simulink model "ofdm_txrx_mimo_2x"  (Simulink 6.6)
settings: Solver=VariableStepDiscrete  StartTime=0.0  StopTime=20e3  RelTol=1e-3
PreLoadFcn:
  | ofdm_rx_mimo_init
InitFcn:
  | ofdm_rx_mimo_init
StartFcn:
  | ofdm_rx_mimo_init
values from neighbouring files (symbols this model uses):
  BER_RAM_init_size = 2048    [ofdm_tx_mimo_init.m]
  CPLength = 16    [ofdm_tx_mimo_init.m]
  CRCPolynomial = hex2dec('04c11db7')    [ofdm_tx_mimo_init.m]
  freqOffset_track_filtCoef_i = hex2dec('2500')/2^32    [ofdm_rx_mimo_init.m]
  freqOffset_track_filtCoef_p = hex2dec('d0000')/2^32    [ofdm_rx_mimo_init.m]
  hi = zeros(1,length(cci))    [ofdm_rx_mimo_init.m]
  hr = zeros(1,length(ccr))    [ofdm_rx_mimo_init.m]
  max_CP_length = 16    [ofdm_tx_mimo_init.m]
  max_num_baseRateSymbols = 31    [ofdm_tx_mimo_init.m]
  max_num_subcarriers = 64    [ofdm_tx_mimo_init.m]
  max_num_trainingSymbols = 15    [ofdm_tx_mimo_init.m]
  max_numBytes = 16384    [ofdm_tx_mimo_init.m]
  max_OFDM_symbols = 2047    [ofdm_tx_mimo_init.m]
  modConstellation_qam16 = .75*[1 3 -1 -3]./3    [ofdm_tx_mimo_init.m]
  modConstellation_qam256 = 0.9375*[3 1 5 7 11 9 13 15 -3 -1 -5 -7 -11 -9 -13 -15]./15    [ofdm_tx_mimo_init.m]
  modConstellation_qam64 = 0.875*[3 1 5 7 -3 -1 -5 -7]./7    [ofdm_tx_mimo_init.m]
  modConstellation_qpsk = [1 -1]./sqrt(2)    [ofdm_tx_mimo_init.m]
  numBaseRateSymbols = 2    [ofdm_tx_mimo_init.m]
  numSubcarriers = 64    [ofdm_tx_mimo_init.m]
  numTrainingSymbols = 2    [ofdm_tx_mimo_init.m]
  pilot1_indicies = 7 + ( (64-7) * 2^16)    (= 3735559)    [ofdm_tx_mimo_init.m]
  pilot2_indicies = 21 + ( (64-21) * 2^16)    (= 2818069)    [ofdm_tx_mimo_init.m]
  pilotValue_neg = hex2dec('8000') + (2^16 * 0)    [ofdm_tx_mimo_init.m]
  pilotValue_pos = hex2dec('7FFF') + (2^16 * 0)    [ofdm_tx_mimo_init.m]
  pkt_crossCorr_thresh = 0.7    [ofdm_rx_mimo_init.m]
  pkt_energy_thresh = 0    [ofdm_rx_mimo_init.m]
  pkt_numPayloadBytes = 1400+42-6    (= 1436)    [ofdm_tx_mimo_init.m]
  pktByteNums = numHeaderBytes +  				byteIndex_numPayloadBytes(1)*2^8 +  				byteIndex_numPayloadBytes(2)*2^16 +  				byteIndex_simpleDynModMasks * 2^24    [ofdm_rx_mimo_init.m]
  PktDet_Delay = 26 + Symbol_Timing_Offset*2^7    (= 1050)    [ofdm_rx_mimo_init.m]
  preamble = 6*[0 shortsyms_10 longsyms_2]    [ofdm_tx_mimo_init.m]
  preamble_I = real(preamble)    [ofdm_tx_mimo_init.m]
  preamble_Q = imag(preamble)    [ofdm_tx_mimo_init.m]
  RAM_init_size = max_numBytes    (= 16384)    [ofdm_tx_mimo_init.m]
  reg_Interrupt_PktBuf_Control = reg_InterruptControl +  	1 * 2^16 +  	0 * 2^24    (= 65656)    [ofdm_rx_mimo_init.m]
  rx_controlBits = 1 * 2  		 + 1 * 4  		 + 0 * 8  		 + 1 * 16 * rx_SISO_Mode  		 + 1 * 32  		 + 1 * 64  		 + 0 * 128  		 + 1 * 256  		 + 0 * 512  		 + 1 * 1024  		 + 0 * 2048  		 + 1 * 4096  		 + 1 * 8192  		 + 0 * 16384  		 + 1 * 65536  		 + 1 * 131072  		 + 0 * 262144  		 + 1 * 524288    [ofdm_rx_mimo_init.m]
  Rx_PhaseNoiseTrack_K = 0.6094    [ofdm_rx_mimo_init.m]
  rx_postEq_scaling = round(rxScaling*2^11) * (1 + 2^16)    [ofdm_rx_mimo_init.m]
  RxDataScrambling_Seq = [40 198 78 63 82 173 102 245 48 111 172 115 147 230 216 93 72 65 62 2 205 242 122 90 128 83 105 97 73 10 5 252]    [ofdm_tx_mimo_init.m]
  simOnly_numSamples = length(preamble)+( (numSubcarriers+CPLength)*(numTrainingSymbols + numBaseRateSymbols + numFullRateSymbols/2) )    [ofdm_tx_mimo_init.m]
  train = [train train]    [ofdm_tx_mimo_init.m]
  tx_controlBits = (antB_preambleShift * 2^4) + (2^0 * tx_SISO_Mode) + 4*0 + 8*1    (= 73)    [ofdm_tx_mimo_init.m]
  TxDataScrambling_Seq = [40 198 78 63 82 173 102 245 48 111 172 115 147 230 216 93 72 65 62 2 205 242 122 90 128 83 105 97 73 10 5 252]    [ofdm_tx_mimo_init.m]
  txReg_symbolCounts = (2^16 * numFullRateSymbols) + (2^8 *numBaseRateSymbols ) + numTrainingSymbols    [ofdm_tx_mimo_init.m]
  TxRx_FFTScaling = bin2dec('010111') + (bin2dec('000101') * 2^6)    [ofdm_tx_mimo_init.m]
undefined symbols (not defined in the file or in its folder): AntA, AntB, BaseRate, CRC_table_gen, gcbh, intfilt, pktTiming_count_payloadStart, pktTiming_symbol_length, xlBlockLoadCallback, xlGetSimulinkPeriod
libraries: xbsIndex_r4 x3973, dspsigops x4, commchan3 x2, commrflib2 x2, dspsrcs4 x1
(part 16 of 38)

== system ofdm_txrx_mimo_2x/OFDM Rx MIMO/Equalizer & Packetizer/Pilot_Extractor/Phase Calc ==
  I : Inport
  Q : Inport  Port=2
  CORDIC ATAN : Subsystem  mask(Xilinx CORDIC ATAN){stages=11, pe_nbits=19, pe_binpt=15, pipeline_x=ones(1,11), prev_stages=11, pipeline=[1  1  1  1  1  1  1  1  1  1  1]}
      mask prompts: stages "Number of Processing Elements (integer value starting from 1)"; pe_nbits "X, Y Data Width"; pe_binpt "X, Y Binary Point Position"; pipeline_x "Latency for each Processing Element [1 0 0 1]"; prev_stages "Previous Number of Stages"; pipeline "Previous Pipeline Values"
      mask help: A parallel implementation, circular vectoring mode CORDIC processor for performing rectangular-to-polar conversion. The number of iteration stages (and resultant output accuracy) and the processor arithmetic precision are controlled by the mask customization.
      mask init: same as ofdm_txrx_mimo_2x/OFDM Rx MIMO/Coarse Freq Correction/Coarse Freq Offset Calc Combo/Arctan2/CORDIC ATAN1
      settings: CopyFcn=set_param(gcb, 'MaskSelfModifiable', 'on', 'LinkStatus', 'none');
      mask Help: eval('xlWeb(xlhtmldoclink(''CORDIC ATAN''))');
      mask Display:
      | patch([0 iWidth iWidth 0],[0 0 iHeight iHeight] , bg);
      | patch(logoX,logoY , fg);
      | text(iCx-6,iCy,'z');
      | text(iCx,iCy+4,str);
      | port_label('input',1,'x');
      | port_label('input',2,'y');
      | port_label('output',1,'mag');
      | port_label('output',2,'atan');
      | plot([0 0 iWidth iWidth 0], [0 iHeight iHeight 0 0]);
  Down Sample : xbsIndex_r4/Down Sample  sample_ratio=2  sample_phase="Last Value of Frame  (most efficient)"  en=off  latency=1  dbl_ovrd=off  block_type=dsamp
      settings: block_version=8.2.02
  Mux : xbsIndex_r4/Mux  inputs=2  en=off  latency=0  precision=Full  arith_type="Signed  (2's comp)"  n_bits=8  bin_pt=2  quantization=Truncate  overflow=Wrap  dbl_ovrd=off  block_type=mux
      settings: xl_area=[16 0 0 32 0 0 0]  block_version=VER_STRING_GOES_HERE
  Up Sample : xbsIndex_r4/Up Sample  sample_ratio=2  copy_samples=on  dbl_ovrd=off  block_type=usamp
      settings: infoedit="Up samples input data.  Inserted values  can be zeros or copies of the most recent input sample.<P><P>Hardware notes: No hardware is needed if inserted values are copies of the input sample; otherwise, a mux and single bit flip-flop are used."  block_version=8.2.02
  Up Sample1 : xbsIndex_r4/Up Sample  sample_ratio=2  copy_samples=on  dbl_ovrd=off  block_type=usamp
      settings: infoedit="Up samples input data.  Inserted values  can be zeros or copies of the most recent input sample.<P><P>Hardware notes: No hardware is needed if inserted values are copies of the input sample; otherwise, a mux and single bit flip-flop are used."  block_version=8.2.02
  Phase : Outport
  wires:
  Up Sample/1 -> CORDIC ATAN/1(x)
  CORDIC ATAN/2(Z) -> Down Sample/1
  Mux/1 -> Phase/1
  [regRx_usePilotArctan] -> Mux/1
  I/1 -> Up Sample/1
  Q/1 -> Mux/2, Up Sample1/1
  Down Sample/1 -> Mux/3
  Up Sample1/1 -> CORDIC ATAN/2(y)

== system ofdm_txrx_mimo_2x/OFDM Rx MIMO/Equalizer & Packetizer/Pilot_Extractor/Pilot_Descrambler ==
  Sym Index : Inport
  rst : Inport  Port=2
  "I " : Inport  Port=3
  "Q " : Inport  Port=4
  FFT Index : Inport  Port=5
  Neg Pilot : Inport  Port=6
  Constant5 : xbsIndex_r4/Constant  arith_type=Unsigned  const=numSubcarriers-1  n_bits=ceil(log2(numSubcarriers))  bin_pt=0  explicit_period=off  period=2  equ=P=C  opselect=C  inp2=PCIN>>17  opr=+  inp1=P  carry=CIN  dbl_ovrd=off  block_type=constant
      settings: block_version=VER_STRING_GOES_HERE
  Gateway Out{1..6} (6 alike) : xbsIndex_r4/Gateway Out  hdl_port=off  timing_constraint=None  locs_specified=off  LOCs={}  block_type=gatewayout
      settings: infoedit="Gateway out block.  Converts Xilinx fixed point inputs into ouputs of type Simulink integer, double, or fixed point.<P><P>Hardware notes:  In hardware these blocks become top level output ports or are discarded, depending on how they are configured."  block_version=VER_STRING_GOES_HERE
  Inverter : xbsIndex_r4/Inverter  en=off  latency=0  dbl_ovrd=off  block_type=inv
      settings: block_version=VER_STRING_GOES_HERE
  LSB : xbsIndex_r4/Slice  nbits=1  boolean_output=on  mode=Lower Bit Location + Width  bit1=0  base1=MSB of Input  bit0=0  base0=LSB of Input  dbl_ovrd=off  block_type=slice
      settings: infoedit="Extracts a given range of bits from each input sample and presents it at the output.  The output type is ordinarily unsigned with binary point at zero, but can be Boolean when the slice is one bit wide.<P><P>Hardware notes: In hardware this block costs nothing."  block_version=VER_STRING_GOES_HERE
  Logical : xbsIndex_r4/Logical  logical_function=XOR  inputs=2  en=off  latency=0  precision=Full  arith_type=Unsigned  n_bits=8  bin_pt=2  align_bp=on  dbl_ovrd=off  block_type=logical
      settings: xl_area=[2 0 0 2 0 0 0]  block_version=VER_STRING_GOES_HERE
  Logical1 : xbsIndex_r4/Logical  logical_function=AND  inputs=3  en=off  latency=0  precision=Full  arith_type=Unsigned  n_bits=8  bin_pt=2  align_bp=on  dbl_ovrd=off  block_type=logical
      settings: xl_area=[2 0 0 2 0 0 0]  block_version=VER_STRING_GOES_HERE
  Mux : xbsIndex_r4/Mux  inputs=2  en=off  latency=0  precision=Full  arith_type="Signed  (2's comp)"  n_bits=8  bin_pt=2  quantization=Truncate  overflow=Wrap  dbl_ovrd=off  block_type=mux
      settings: xl_area=[16 0 0 32 0 0 0]  block_version=VER_STRING_GOES_HERE
  Mux1 : xbsIndex_r4/Mux  inputs=2  en=off  latency=0  precision=Full  arith_type="Signed  (2's comp)"  n_bits=8  bin_pt=2  quantization=Truncate  overflow=Wrap  dbl_ovrd=off  block_type=mux
      settings: xl_area=[16 0 0 32 0 0 0]  block_version=VER_STRING_GOES_HERE
  Negate : xbsIndex_r4/Negate  precision=User Defined  arith_type="Signed  (2's comp)"  n_bits=17  bin_pt=15  quantization="Round  (unbiased: +/- Inf)"  overflow=Saturate  en=off  latency=0  dbl_ovrd=off  block_type=negate
      settings: block_version=VER_STRING_GOES_HERE
  Negate1 : xbsIndex_r4/Negate  precision=User Defined  arith_type="Signed  (2's comp)"  n_bits=17  bin_pt=15  quantization="Round  (unbiased: +/- Inf)"  overflow=Saturate  en=off  latency=0  dbl_ovrd=off  block_type=negate
      settings: block_version=VER_STRING_GOES_HERE
  Pilot Scrambling Sequence Gen : Subsystem
  Relational2 : xbsIndex_r4/Relational  mode=a=b  en=off  latency=0  dbl_ovrd=off  block_type=relational
      settings: xl_area=[6 0 0 11 0 0 0]  block_version=VER_STRING_GOES_HERE
  Relational3 : xbsIndex_r4/Relational  mode=a>=b  en=off  latency=0  dbl_ovrd=off  block_type=relational
      settings: xl_area=[6 0 0 11 0 0 0]  block_version=VER_STRING_GOES_HERE
  Rx Pilot Descrambling : Scope  NumInputPorts=6
      settings: YMin=-1~0.95~0.95~-1~-0.1~-0.075  YMax=1~1.05~1.05~1~0.1~0.1  SaveName=ScopeData41
  S-R Latch : Subsystem
  Shift : xbsIndex_r4/Shift  shift_dir=Left  shift_bits=1  en=off  latency=0  precision=User Defined  arith_type=Unsigned  n_bits=1+ceil(log2(max_num_trainingSymbols))  bin_pt=0  quantization=Truncate  overflow=Flag as error  dbl_ovrd=off  block_type=shift
      settings: block_version=VER_STRING_GOES_HERE
  posedge : Subsystem
  posedge1 : Subsystem
  I : Outport
  Q : Outport  Port=2
  wires:
  Constant5/1 -> Relational2/1
  rst/1 -> Pilot Scrambling Sequence Gen/2(rst), Gateway Out5/1, S-R Latch/2(R)
  "I "/1 -> Mux/2, Gateway Out6/1, Negate/1
  "Q "/1 -> Negate1/1, Mux1/2
  Pilot Scrambling Sequence Gen/1(Q) -> Gateway Out3/1, Logical/1
  Neg Pilot/1 -> Logical/2
  FFT Index/1 -> Relational2/2
  Mux/1 -> I/1, Gateway Out1/1
  Mux1/1 -> Q/1
  Sym Index/1 -> Relational3/1, LSB/1
  Shift/1 -> Relational3/2
  Logical1/1 -> Pilot Scrambling Sequence Gen/1(en), Gateway Out2/1
  Relational2/1 -> posedge1/1(D)
  Relational3/1 -> Gateway Out4/1, posedge/1(D)
  Gateway Out5/1 -> Rx Pilot Descrambling/1
  Gateway Out4/1 -> Rx Pilot Descrambling/2
  Gateway Out3/1 -> Rx Pilot Descrambling/3
  Gateway Out2/1 -> Rx Pilot Descrambling/4
  [regRx_numTrainingSymbols] -> Shift/1
  Logical/1 -> Mux1/1, Mux/1
  Gateway Out6/1 -> Rx Pilot Descrambling/5
  Gateway Out1/1 -> Rx Pilot Descrambling/6
  posedge/1(Q) -> S-R Latch/1(S)
  S-R Latch/1(D) -> Logical1/3
  Negate/1 -> Mux/3
  Negate1/1 -> Mux1/3
  LSB/1 -> Inverter/1
  Inverter/1 -> Logical1/1
  posedge1/1(Q) -> Logical1/2

== system ofdm_txrx_mimo_2x/OFDM Rx MIMO/Equalizer & Packetizer/Pilot_Extractor/S-R Latch ==
  S : Inport
  R : Inport  Port=2
  Constant2 : xbsIndex_r4/Constant  arith_type=Boolean  const=1  n_bits=1  bin_pt=0  explicit_period=off  period=1  equ=P=C  opselect=C  inp2=PCIN>>17  opr=+  inp1=P  carry=CIN  dbl_ovrd=off  block_type=constant
      settings: block_version=VER_STRING_GOES_HERE
  Register : xbsIndex_r4/Register  init=0  rst=on  en=on  dbl_ovrd=off  block_type=register
      settings: xl_area=[0, 0, 0, 0, 0, 0, 0]  block_version=VER_STRING_GOES_HERE
  D : Outport
  wires:
  Constant2/1 -> Register/1
  R/1 -> Register/2
  S/1 -> Register/3
  Register/1 -> D/1

== system ofdm_txrx_mimo_2x/OFDM Rx MIMO/Equalizer & Packetizer/Pilot_Extractor/posedge ==
  D : Inport  Orientation=down
  Inverter : xbsIndex_r4/Inverter  en=off  latency=1  dbl_ovrd=off  block_type=inv
      settings: block_version=VER_STRING_GOES_HERE
  Logical1 : xbsIndex_r4/Logical  logical_function=AND  inputs=2  en=off  latency=0  precision=Full  arith_type=Unsigned  n_bits=8  bin_pt=2  align_bp=on  dbl_ovrd=off  block_type=logical
      settings: xl_area=[1 0 0 1 0 0 0]  block_version=VER_STRING_GOES_HERE
  Q : Outport
  wires:
  Inverter/1 -> Logical1/2
  D/1 -> Logical1/1, Inverter/1
  Logical1/1 -> Q/1

== system ofdm_txrx_mimo_2x/OFDM Rx MIMO/FFT & Chan Est/Addr Gen1/Copy_Downsample ==
  D : Inport
  Delay : xbsIndex_r4/Delay  en=off  latency=1  dbl_ovrd=off  reg_retiming=off  block_type=delay
      settings: infoedit=Hardware notes: A delay line is a chain, each link of which is an SRL16 followed by a flip-flop. If register retiming is enabled, the delay line is a chain of flip-flops.  block_version=VER_STRING_GOES_HERE
  Down Sample : xbsIndex_r4/Down Sample  sample_ratio=2  sample_phase="Last Value of Frame  (most efficient)"  en=off  latency=1  dbl_ovrd=off  block_type=dsamp
      settings: block_version=VER_STRING_GOES_HERE
  Logical : xbsIndex_r4/Logical  logical_function=OR  inputs=2  en=off  latency=0  precision=Full  arith_type=Unsigned  n_bits=16  bin_pt=0  align_bp=on  dbl_ovrd=off  block_type=logical
      settings: block_version=VER_STRING_GOES_HERE
  Q : Outport
  wires:
  Delay/1 -> Logical/1
  D/1 -> Delay/1, Logical/2
  Logical/1 -> Down Sample/1
  Down Sample/1 -> Q/1

== system ofdm_txrx_mimo_2x/OFDM Rx MIMO/FFT & Chan Est/Addr Gen1/Oscillator ==
  en : Inport
  rst : Inport  Port=2
  Assert : xbsIndex_r4/Assert  Orientation=left  assert_type=on  type_source=Explicitly  arith_type=Boolean  n_bits=16  bin_pt=14  assert_rate=on  rate_source=Explicitly  period=2  output_port=on  block_type=assert
      settings: infoedit=Asserts a user-defined sample rate and/or type on a signal.<P><P>Hardware notes: In hardware this block costs nothing.  block_version=VER_STRING_GOES_HERE
  Inverter1 : xbsIndex_r4/Inverter  Orientation=left  en=off  latency=0  dbl_ovrd=off  block_type=inv
      settings: block_version=VER_STRING_GOES_HERE
  Register : xbsIndex_r4/Register  init=0  rst=on  en=on  dbl_ovrd=off  block_type=register
      settings: block_version=VER_STRING_GOES_HERE
  Q : Outport
  wires:
  Register/1 -> Q/1, Assert/1
  en/1 -> Register/3
  Inverter1/1 -> Register/1
  rst/1 -> Register/2
  Assert/1 -> Inverter1/1

== system ofdm_txrx_mimo_2x/OFDM Rx MIMO/FFT & Chan Est/Addr Gen1/Output Sym Counter ==
  FFT Done : Inport
  Not Training : Inport  Port=2
  Payload : Inport  Port=3
  Read Addr : Inport  Port=4
  Constant1 : xbsIndex_r4/Constant  arith_type=Unsigned  const=numSubcarriers-1  n_bits=ceil(log2(numSubcarriers))  bin_pt=0  explicit_period=off  period=1  equ=P=C  opselect=C  inp2=PCIN>>17  opr=+  inp1=P  carry=CIN  dbl_ovrd=off  block_type=constant
      settings: block_version=VER_STRING_GOES_HERE
  Counter1 : xbsIndex_r4/Counter  cnt_type=Free Running  cnt_to=127  operation=Up  start_count=0  cnt_by_val=1  arith_type=Unsigned  n_bits=ceil(log2(max_OFDM_symbols))  bin_pt=0  load_pin=off  rst=on  en=on  explicit_period=off  period=2  dbl_ovrd=off  use_behavioral_HDL=off  use_rpm=on  implementation=Fabric  block_type=counter
      settings: xl_area=[6 12 0 12 0 0 0]  block_version=VER_STRING_GOES_HERE
  FFT Output Buffers Addrs 3 : Scope  NumInputPorts=6
      settings: TimeRange=4000  YMin=5~0~0~-1~0~0  YMax=13~1~1~1~1~65  SaveName=ScopeData75
  Inverter3 : xbsIndex_r4/Inverter  en=off  latency=0  dbl_ovrd=off  block_type=inv
      settings: block_version=VER_STRING_GOES_HERE
  Logical1 : xbsIndex_r4/Logical  logical_function=AND  inputs=2  en=off  latency=0  precision=Full  arith_type=Unsigned  n_bits=8  bin_pt=2  align_bp=on  dbl_ovrd=off  block_type=logical
      settings: xl_area=[0, 0, 0, 0, 0, 0, 0]  block_version=VER_STRING_GOES_HERE
  Logical2 : xbsIndex_r4/Logical  logical_function=OR  inputs=2  en=off  latency=0  precision=Full  arith_type=Unsigned  n_bits=8  bin_pt=2  align_bp=on  dbl_ovrd=off  block_type=logical
      settings: xl_area=[0, 0, 0, 0, 0, 0, 0]  block_version=VER_STRING_GOES_HERE
  Logical3 : xbsIndex_r4/Logical  logical_function=AND  inputs=2  en=off  latency=0  precision=Full  arith_type=Unsigned  n_bits=8  bin_pt=2  align_bp=on  dbl_ovrd=off  block_type=logical
      settings: xl_area=[0, 0, 0, 0, 0, 0, 0]  block_version=VER_STRING_GOES_HERE
  Logical4 : xbsIndex_r4/Logical  logical_function=AND  inputs=2  en=off  latency=0  precision=Full  arith_type=Unsigned  n_bits=8  bin_pt=2  align_bp=on  dbl_ovrd=off  block_type=logical
      settings: xl_area=[0, 0, 0, 0, 0, 0, 0]  block_version=VER_STRING_GOES_HERE
  Posedge : Subsystem
  Posedge1 : Subsystem
  Posedge2 : Subsystem
  Posedge3 : Subsystem
  Relational : xbsIndex_r4/Relational  mode=a=b  en=off  latency=0  dbl_ovrd=off  block_type=relational
      settings: block_version=VER_STRING_GOES_HERE
  S-R Latch2 : Subsystem
  done{1,9..10,16..18} (6 alike) : xbsIndex_r4/Gateway Out  hdl_port=off  timing_constraint=None  locs_specified=off  LOCs={}  block_type=gatewayout
      settings: infoedit="Gateway out block.  Converts Xilinx fixed point inputs into ouputs of type Simulink integer, double, or fixed point.<P><P>Hardware notes:  In hardware these blocks become top level output ports or are discarded, depending on how they are configured."  xl_area=[0 0 0 0 1 0 0]  block_version=VER_STRING_GOES_HERE
  Sym Index : Outport
  wires:
  Logical4/1 -> Logical3/2
  S-R Latch2/1(D) -> Logical4/2
  Posedge/1(Q) -> S-R Latch2/1(S), Logical4/1, done16/1
  FFT Done/1 -> Posedge/1(D)
  Counter1/1 -> Sym Index/1, done9/1
  Not Training/1 -> Inverter3/1, Logical1/2
  Inverter3/1 -> Logical3/1
  Payload/1 -> Posedge1/1(D)
  Posedge1/1(Q) -> S-R Latch2/2(R), Counter1/1
  Read Addr/1 -> Relational/2, done1/1
  Constant1/1 -> Relational/1
  Relational/1 -> Logical1/1
  Logical1/1 -> Posedge2/1(D)
  Logical3/1 -> Posedge3/1(D)
  Logical2/1 -> Counter1/2, done10/1
  done16/1 -> FFT Output Buffers Addrs 3/5
  done9/1 -> FFT Output Buffers Addrs 3/1
  done10/1 -> FFT Output Buffers Addrs 3/2
  done18/1 -> FFT Output Buffers Addrs 3/3
  done17/1 -> FFT Output Buffers Addrs 3/4
  done1/1 -> FFT Output Buffers Addrs 3/6
  Posedge2/1(Q) -> Logical2/1, done18/1
  Posedge3/1(Q) -> Logical2/2, done17/1

== system ofdm_txrx_mimo_2x/OFDM Rx MIMO/FFT & Chan Est/Addr Gen1/S-R Latch1 ==
  S : Inport
  R : Inport  Port=2
  Constant2 : xbsIndex_r4/Constant  arith_type=Boolean  const=1  n_bits=1  bin_pt=0  explicit_period=off  period=1  equ=P=C  opselect=C  inp2=PCIN>>17  opr=+  inp1=P  carry=CIN  dbl_ovrd=off  block_type=constant
      settings: block_version=VER_STRING_GOES_HERE
  Register : xbsIndex_r4/Register  init=0  rst=on  en=on  dbl_ovrd=off  block_type=register
      settings: xl_area=[0, 0, 0, 0, 0, 0, 0]  block_version=VER_STRING_GOES_HERE
  D : Outport
  wires:
  Register/1 -> D/1
  S/1 -> Register/3
  R/1 -> Register/2
  Constant2/1 -> Register/1

== system ofdm_txrx_mimo_2x/OFDM Rx MIMO/FFT & Chan Est/Addr_Generator/Initial Read Addr Timing Calc ==
  note: The FFT runs 4x as fast as the input samples. As the input
      | samples are written to the buffers, the FFT waits to start
      | until it can have access to the newest sample it needs
      | 1 cycle after it's written.
  AddSub1 : xbsIndex_r4/AddSub  mode=Addition  use_carryin=off  use_carryout=off  en=off  latency=0  precision=User Defined  arith_type=Unsigned  n_bits=ceil(log2(max_num_subcarriers))+1  bin_pt=0  quantization=Truncate  overflow=Wrap  dbl_ovrd=off  use_behavioral_HDL=on  pipelined=off  use_rpm=on  hw_selection=Fabric  block_type=addsub
      settings: xl_area=[4 0 0 6 0 0 0]  block_version=VER_STRING_GOES_HERE
  Constant1 : xbsIndex_r4/Constant  arith_type=Unsigned  const=0.75*numSubcarriers  n_bits=log2(numSubcarriers)  bin_pt=0  explicit_period=off  period=1  equ=P=C  opselect=C  inp2=PCIN>>17  opr=+  inp1=P  carry=CIN  dbl_ovrd=off  block_type=constant
      settings: block_version=VER_STRING_GOES_HERE
  Display : Display
  Display1 : Display
  Display3 : Display
  Up Sample4 : xbsIndex_r4/Up Sample  sample_ratio=2  copy_samples=on  dbl_ovrd=off  block_type=usamp
      settings: infoedit="Up samples input data.  Inserted values  can be zeros or copies of the most recent input sample.<P><P>Hardware notes: No hardware is needed if inserted values are copies of the input sample; otherwise, a mux and single bit flip-flop are used."  block_version=VER_STRING_GOES_HERE
  done2 : xbsIndex_r4/Gateway Out  hdl_port=off  timing_constraint=None  locs_specified=off  LOCs={}  block_type=gatewayout
      settings: infoedit="Gateway out block.  Converts Xilinx fixed point inputs into ouputs of type Simulink integer, double, or fixed point.<P><P>Hardware notes:  In hardware these blocks become top level output ports or are discarded, depending on how they are configured."  xl_area=[0 0 0 0 1 0 0]  block_version=VER_STRING_GOES_HERE
  done7 : xbsIndex_r4/Gateway Out  hdl_port=off  timing_constraint=None  locs_specified=off  LOCs={}  block_type=gatewayout
      settings: infoedit="Gateway out block.  Converts Xilinx fixed point inputs into ouputs of type Simulink integer, double, or fixed point.<P><P>Hardware notes:  In hardware these blocks become top level output ports or are discarded, depending on how they are configured."  xl_area=[0 0 0 0 1 0 0]  block_version=VER_STRING_GOES_HERE
  done9 : xbsIndex_r4/Gateway Out  hdl_port=off  timing_constraint=None  locs_specified=off  LOCs={}  block_type=gatewayout
      settings: infoedit="Gateway out block.  Converts Xilinx fixed point inputs into ouputs of type Simulink integer, double, or fixed point.<P><P>Hardware notes:  In hardware these blocks become top level output ports or are discarded, depending on how they are configured."  xl_area=[0 0 0 0 1 0 0]  block_version=VER_STRING_GOES_HERE
  D : Outport
  wires:
  AddSub1/1 -> done2/1, D/1
  done9/1 -> Display3/1
  done7/1 -> Display1/1
  done2/1 -> Display/1
  [regRx_symbolTimingOffset] -> Up Sample4/1
  Up Sample4/1 -> done9/1, AddSub1/2
  Constant1/1 -> done7/1, AddSub1/1

== system ofdm_txrx_mimo_2x/OFDM Rx MIMO/FFT & Chan Est/Addr_Generator/Posedge ==
  D : Inport
  Delay : xbsIndex_r4/Delay  en=off  latency=1  dbl_ovrd=off  reg_retiming=off  block_type=delay
      settings: infoedit=Hardware notes: A delay line is a chain, each link of which is an SRL16 followed by a flip-flop. If register retiming is enabled, the delay line is a chain of flip-flops.  xl_area=[1 1 0 0 0 0 0]  block_version=VER_STRING_GOES_HERE
  Inverter : xbsIndex_r4/Inverter  en=off  latency=0  dbl_ovrd=off  block_type=inv
      settings: xl_area=[0 0 0 0 0 0 0]  block_version=VER_STRING_GOES_HERE
  Logical : xbsIndex_r4/Logical  logical_function=AND  inputs=2  en=off  latency=0  precision=Full  arith_type=Unsigned  n_bits=8  bin_pt=2  align_bp=on  dbl_ovrd=off  block_type=logical
      settings: xl_area=[1 0 0 1 0 0 0]  block_version=VER_STRING_GOES_HERE
  Q : Outport
  wires:
  Logical/1 -> Q/1
  D/1 -> Delay/1, Logical/1
  Inverter/1 -> Logical/2
  Delay/1 -> Inverter/1

== system ofdm_txrx_mimo_2x/OFDM Rx MIMO/FFT & Chan Est/Addr_Generator/negedge1 ==
  D : Inport
  Delay : xbsIndex_r4/Delay  en=off  latency=1  dbl_ovrd=off  reg_retiming=off  block_type=delay
      settings: infoedit=Hardware notes: A delay line is a chain, each link of which is an SRL16 followed by a flip-flop. If register retiming is enabled, the delay line is a chain of flip-flops.  xl_area=[1 1 0 0 0 0 0]  block_version=VER_STRING_GOES_HERE
  Inverter : xbsIndex_r4/Inverter  en=off  latency=0  dbl_ovrd=off  block_type=inv
      settings: xl_area=[0 0 0 0 0 0 0]  block_version=VER_STRING_GOES_HERE
  Logical : xbsIndex_r4/Logical  logical_function=AND  inputs=2  en=off  latency=0  precision=Full  arith_type=Unsigned  n_bits=8  bin_pt=2  align_bp=on  dbl_ovrd=off  block_type=logical
      settings: xl_area=[1 0 0 1 0 0 0]  block_version=VER_STRING_GOES_HERE
  Q : Outport
  wires:
  Inverter/1 -> Logical/1
  Delay/1 -> Logical/2
  D/1 -> Inverter/1, Delay/1
  Logical/1 -> Q/1

== system ofdm_txrx_mimo_2x/OFDM Rx MIMO/FFT & Chan Est/Channel Estimation/Bus Creator1 ==
  AA-I : Inport
  AA-Q : Inport  Port=2
  AB-I : Inport  Port=3
  AB-Q : Inport  Port=4
  BA-I : Inport  Port=5
  BA-Q : Inport  Port=6
  BB-I : Inport  Port=7
  BB-Q : Inport  Port=8
  Bus Creator1 : BusCreator
  Bus Creator2 : BusCreator
  I : Outport
  Q : Outport  Port=2
  wires:
  AA-I/1 -> Bus Creator1/1
  AB-I/1 -> Bus Creator1/2
  Bus Creator1/1 -> I/1
  BA-I/1 -> Bus Creator1/3
  BB-I/1 -> Bus Creator1/4
  AA-Q/1 -> Bus Creator2/1
  AB-Q/1 -> Bus Creator2/2
  Bus Creator2/1 -> Q/1
  BA-Q/1 -> Bus Creator2/3
  BB-Q/1 -> Bus Creator2/4

== system ofdm_txrx_mimo_2x/OFDM Rx MIMO/FFT & Chan Est/Channel Estimation/Downsamplng ==
  AA-I : Inport
  AA-Q : Inport  Port=2
  AB-I : Inport  Port=3
  AB-Q : Inport  Port=4
  BA-I : Inport  Port=5
  BA-Q : Inport  Port=6
  BB-I : Inport  Port=7
  BB-Q : Inport  Port=8
  Down Sample{,1..7} (8 alike) : xbsIndex_r4/Down Sample  sample_ratio=2  sample_phase="Last Value of Frame  (most efficient)"  en=off  latency=1  dbl_ovrd=off  block_type=dsamp
      settings: block_version=10.1.2
  " AA-I" : Outport
  " AA-Q" : Outport  Port=2
  " AB-I" : Outport  Port=3
  " AB-Q" : Outport  Port=4
  " BA-I" : Outport  Port=5
  " BA-Q" : Outport  Port=6
  " BB-I" : Outport  Port=7
  " BB-Q" : Outport  Port=8
  wires:
  AA-I/1 -> Down Sample/1
  AB-I/1 -> Down Sample1/1
  AA-Q/1 -> Down Sample4/1
  BA-I/1 -> Down Sample2/1
  AB-Q/1 -> Down Sample5/1
  BB-I/1 -> Down Sample3/1
  BA-Q/1 -> Down Sample6/1
  BB-Q/1 -> Down Sample7/1
  Down Sample/1 -> " AA-I"/1
  Down Sample1/1 -> " AB-I"/1
  Down Sample2/1 -> " BA-I"/1
  Down Sample3/1 -> " BB-I"/1
  Down Sample4/1 -> " AA-Q"/1
  Down Sample5/1 -> " AB-Q"/1
  Down Sample6/1 -> " BA-Q"/1
  Down Sample7/1 -> " BB-Q"/1

== system ofdm_txrx_mimo_2x/OFDM Rx MIMO/FFT & Chan Est/Channel Estimation/H_A-A Estimate Buffer ==
  I : Inport
  Q : Inport  Port=2
  Est_WE : Inport  Port=3
  Est_Reset : Inport  Port=4
  Xk_Index : Inport  Port=5
  I Estimate Buffer : Subsystem
  Q Estimate Buffer : Subsystem
  EstI : Outport
  EstQ : Outport  Port=2
  wires:
  Q/1 -> Q Estimate Buffer/1(Est_Data)
  Q Estimate Buffer/1(h[n]) -> EstQ/1
  Est_Reset/1 -> Q Estimate Buffer/4(Rst), I Estimate Buffer/4(Rst)
  Est_WE/1 -> Q Estimate Buffer/3(Est_WE), I Estimate Buffer/3(Est_WE)
  I Estimate Buffer/1(h[n]) -> EstI/1
  Xk_Index/1 -> Q Estimate Buffer/2(Est_Addr), I Estimate Buffer/2(Est_Addr)
  I/1 -> I Estimate Buffer/1(Est_Data)

== system ofdm_txrx_mimo_2x/OFDM Rx MIMO/FFT & Chan Est/Channel Estimation/H_A-B Estimate Buffer ==
  I : Inport
  Q : Inport  Port=2
  Est_WE : Inport  Port=3
  Est_Reset : Inport  Port=4
  Xk_Index : Inport  Port=5
  I Estimate Buffer : Subsystem
  Q Estimate Buffer : Subsystem
  EstI : Outport
  EstQ : Outport  Port=2
  wires:
  I/1 -> I Estimate Buffer/1(Est_Data)
  Xk_Index/1 -> I Estimate Buffer/2(Est_Addr), Q Estimate Buffer/2(Est_Addr)
  I Estimate Buffer/1(h[n]) -> EstI/1
  Est_WE/1 -> I Estimate Buffer/3(Est_WE), Q Estimate Buffer/3(Est_WE)
  Est_Reset/1 -> I Estimate Buffer/4(Rst), Q Estimate Buffer/4(Rst)
  Q Estimate Buffer/1(h[n]) -> EstQ/1
  Q/1 -> Q Estimate Buffer/1(Est_Data)

== system ofdm_txrx_mimo_2x/OFDM Rx MIMO/FFT & Chan Est/Channel Estimation/H_B-A Estimate Buffer ==
  I : Inport
  Q : Inport  Port=2
  Est_WE : Inport  Port=3
  Est_Reset : Inport  Port=4
  Xk_Index : Inport  Port=5
  I Estimate Buffer : Subsystem
  Q Estimate Buffer : Subsystem
  EstI : Outport
  EstQ : Outport  Port=2
  wires:
  I/1 -> I Estimate Buffer/1(Est_Data)
  Xk_Index/1 -> I Estimate Buffer/2(Est_Addr), Q Estimate Buffer/2(Est_Addr)
  I Estimate Buffer/1(h[n]) -> EstI/1
  Est_WE/1 -> I Estimate Buffer/3(Est_WE), Q Estimate Buffer/3(Est_WE)
  Est_Reset/1 -> I Estimate Buffer/4(Rst), Q Estimate Buffer/4(Rst)
  Q Estimate Buffer/1(h[n]) -> EstQ/1
  Q/1 -> Q Estimate Buffer/1(Est_Data)

== system ofdm_txrx_mimo_2x/OFDM Rx MIMO/FFT & Chan Est/Channel Estimation/H_B-B Estimate Buffer ==
  I : Inport
  Q : Inport  Port=2
  Est_WE : Inport  Port=3
  Est_Reset : Inport  Port=4
  Xk_Index : Inport  Port=5
  I Estimate Buffer : Subsystem
  Q Estimate Buffer : Subsystem
  EstI : Outport
  EstQ : Outport  Port=2
  wires:
  Q/1 -> Q Estimate Buffer/1(Est_Data)
  Q Estimate Buffer/1(h[n]) -> EstQ/1
  Est_Reset/1 -> Q Estimate Buffer/4(Rst), I Estimate Buffer/4(Rst)
  Est_WE/1 -> Q Estimate Buffer/3(Est_WE), I Estimate Buffer/3(Est_WE)
  I Estimate Buffer/1(h[n]) -> EstI/1
  Xk_Index/1 -> Q Estimate Buffer/2(Est_Addr), I Estimate Buffer/2(Est_Addr)
  I/1 -> I Estimate Buffer/1(Est_Data)

== system ofdm_txrx_mimo_2x/OFDM Rx MIMO/FFT & Chan Est/Channel Estimation/Mult by training ==
  AR : Inport
  AI : Inport  Port=2
  BR : Inport  Port=3
  Constant1 : xbsIndex_r4/Constant  arith_type=Signed (2's comp)  const=0  n_bits=2  bin_pt=1  explicit_period=off  period=1  equ=P=C  opselect=C  inp2=PCIN>>17  opr=+  inp1=P  carry=CIN  dbl_ovrd=off  block_type=constant
      settings: block_version=VER_STRING_GOES_HERE
  Constant2 : xbsIndex_r4/Constant  arith_type=Signed (2's comp)  const=0  n_bits=2  bin_pt=1  explicit_period=off  period=1  equ=P=C  opselect=C  inp2=PCIN>>17  opr=+  inp1=P  carry=CIN  dbl_ovrd=off  block_type=constant
      settings: block_version=VER_STRING_GOES_HERE
  Mult by Training : Scope  NumInputPorts=5
      settings: TimeRange=5000  YMin=-1~-1~-1~0~0  YMax=1~1~1~1~1  SaveName=ScopeData22
  Mux3 : xbsIndex_r4/Mux  inputs=3  en=off  latency=1  precision=Full  arith_type="Signed  (2's comp)"  n_bits=8  bin_pt=2  quantization=Truncate  overflow=Wrap  dbl_ovrd=off  block_type=mux
      settings: xl_area=[0, 0, 0, 0, 0, 0, 0]  block_version=VER_STRING_GOES_HERE
  Mux4 : xbsIndex_r4/Mux  inputs=3  en=off  latency=1  precision=Full  arith_type="Signed  (2's comp)"  n_bits=8  bin_pt=2  quantization=Truncate  overflow=Wrap  dbl_ovrd=off  block_type=mux
      settings: xl_area=[0, 0, 0, 0, 0, 0, 0]  block_version=VER_STRING_GOES_HERE
  Negate : xbsIndex_r4/Negate  precision=User Defined  arith_type="Signed  (2's comp)"  n_bits=16  bin_pt=15  quantization=Truncate  overflow=Flag as error  en=off  latency=0  dbl_ovrd=off  block_type=negate
      settings: xl_area=[0, 0, 0, 0, 0, 0, 0]  block_version=VER_STRING_GOES_HERE
  Negate1 : xbsIndex_r4/Negate  precision=User Defined  arith_type="Signed  (2's comp)"  n_bits=16  bin_pt=15  quantization=Truncate  overflow=Flag as error  en=off  latency=0  dbl_ovrd=off  block_type=negate
      settings: xl_area=[0, 0, 0, 0, 0, 0, 0]  block_version=VER_STRING_GOES_HERE
  Reinterpret : xbsIndex_r4/Reinterpret  force_arith_type=on  arith_type=Unsigned  force_bin_pt=on  bin_pt=0  block_type=cast
      settings: infoedit="Changes type of samples without altering their binary representation.<P><P>Hardware notes: In hardware this block costs nothing.<P><P>Example:  Suppose the input is 6 bits wide, signed, with 2 fractional bits, and the output is forced to unsigned with 0 fractional bits.  Then an input of -2.0 (1110.00 in binary 2's complement) becomes an output of 56 (111000 in binary)."  block_version=VER_STRING_GOES_HERE
  done{1..2,7,12..13} (5 alike) : xbsIndex_r4/Gateway Out  hdl_port=off  timing_constraint=None  locs_specified=off  LOCs={}  block_type=gatewayout
      settings: infoedit="Gateway out block.  Converts Xilinx fixed point inputs into ouputs of type Simulink integer, double, or fixed point.<P><P>Hardware notes:  In hardware these blocks become top level output ports or are discarded, depending on how they are configured."  xl_area=[0 0 0 0 1 0 0]  block_version=VER_STRING_GOES_HERE
  PR : Outport
  PI : Outport  Port=2
  wires:
  done1/1 -> Mult by Training/5
  done7/1 -> Mult by Training/4
  done2/1 -> Mult by Training/1
  done13/1 -> Mult by Training/3
  done12/1 -> Mult by Training/2
  Reinterpret/1 -> Mux4/1, Mux3/1
  Mux3/1 -> done7/1, PR/1
  Negate/1 -> Mux3/4
  Constant2/1 -> Mux3/2
  Mux4/1 -> done1/1, PI/1
  Negate1/1 -> Mux4/4
  Constant1/1 -> Mux4/2
  AI/1 -> done13/1, Mux4/3, Negate1/1
  BR/1 -> done2/1, Reinterpret/1
  AR/1 -> done12/1, Mux3/3, Negate/1

== system ofdm_txrx_mimo_2x/OFDM Rx MIMO/FFT & Chan Est/Channel Estimation/WE Gen ==
  note: SISOMode=1 writes estimates for antenna A for every
      | training symbol. SISOMode=0 (i.e. MIMO mode) alternates
      | between antenna A and B training symbols.
  Vin : Inport
  Sym Index : Inport  Port=2
  Train : Inport  Port=3
  1LSB : xbsIndex_r4/Slice  nbits=1  boolean_output=on  mode=Lower Bit Location + Width  bit1=0  base1=MSB of Input  bit0=0  base0=LSB of Input  dbl_ovrd=off  block_type=slice
      settings: infoedit="Extracts a given range of bits from each input sample and presents it at the output.  The output type is ordinarily unsigned with binary point at zero, but can be Boolean when the slice is one bit wide.<P><P>Hardware notes: In hardware this block costs nothing."  block_version=VER_STRING_GOES_HERE
  1LSB+1 : xbsIndex_r4/Slice  nbits=1  boolean_output=on  mode=Lower Bit Location + Width  bit1=0  base1=MSB of Input  bit0=1  base0=LSB of Input  dbl_ovrd=off  block_type=slice
      settings: infoedit="Extracts a given range of bits from each input sample and presents it at the output.  The output type is ordinarily unsigned with binary point at zero, but can be Boolean when the slice is one bit wide.<P><P>Hardware notes: In hardware this block costs nothing."  block_version=VER_STRING_GOES_HERE
  Delay{1..4} (4 alike) : xbsIndex_r4/Delay  en=off  latency=1  dbl_ovrd=off  reg_retiming=on  block_type=delay
      settings: infoedit=Hardware notes: A delay line is a chain, each link of which is an SRL16 followed by a flip-flop. If register retiming is enabled, the delay line is a chain of flip-flops.  xl_area=[0, 0, 0, 0, 0, 0, 0]  block_version=VER_STRING_GOES_HERE
  Expression : xbsIndex_r4/Expression  expression=a & b & ~c & ~d  align_bp=on  en=off  latency=0  precision=Full  arith_type=Unsigned  n_bits=16  bin_pt=0  dbl_ovrd=off  block_type=expr
      settings: block_version=VER_STRING_GOES_HERE
  Expression10 : xbsIndex_r4/Expression  expression=a & b & c & d  align_bp=on  en=off  latency=0  precision=Full  arith_type=Unsigned  n_bits=16  bin_pt=0  dbl_ovrd=off  block_type=expr
      settings: block_version=VER_STRING_GOES_HERE
  Expression8 : xbsIndex_r4/Expression  expression=a & b & ~c & d  align_bp=on  en=off  latency=0  precision=Full  arith_type=Unsigned  n_bits=16  bin_pt=0  dbl_ovrd=off  block_type=expr
      settings: block_version=VER_STRING_GOES_HERE
  Expression9 : xbsIndex_r4/Expression  expression=a & b & c & ~d  align_bp=on  en=off  latency=0  precision=Full  arith_type=Unsigned  n_bits=16  bin_pt=0  dbl_ovrd=off  block_type=expr
      settings: block_version=VER_STRING_GOES_HERE
  Inverter : xbsIndex_r4/Inverter  en=off  latency=0  dbl_ovrd=off  block_type=inv
      settings: block_version=VER_STRING_GOES_HERE
  Logical2 : xbsIndex_r4/Logical  logical_function=AND  inputs=2  en=off  latency=0  precision=Full  arith_type=Unsigned  n_bits=8  bin_pt=2  align_bp=on  dbl_ovrd=off  block_type=logical
      settings: xl_area=[1 0 0 1 0 0 0]  block_version=VER_STRING_GOES_HERE
  Seq0 : Outport
  Seq1 : Outport  Port=2
  Seq2 : Outport  Port=3
  Seq3 : Outport  Port=4
  wires:
  Inverter/1 -> Logical2/1
  1LSB+1/1 -> Logical2/2
  [regRx_SISOmode] -> Inverter/1
  Delay4/1 -> Seq3/1
  Delay2/1 -> Seq2/1
  Delay1/1 -> Seq1/1
  Delay3/1 -> Seq0/1
  1LSB/1 -> Expression10/4, Expression9/4, Expression8/4, Expression/4
  Vin/1 -> Expression10/1, Expression9/1, Expression8/1, Expression/1
  Expression10/1 -> Delay4/1
  Expression9/1 -> Delay2/1
  Expression8/1 -> Delay1/1
  Expression/1 -> Delay3/1
  Logical2/1 -> Expression/3, Expression8/3, Expression9/3, Expression10/3
  Sym Index/1 -> 1LSB/1, 1LSB+1/1
  Train/1 -> Expression/2, Expression8/2, Expression9/2, Expression10/2

== system ofdm_txrx_mimo_2x/OFDM Rx MIMO/FFT & Chan Est/Input Buffers/Antenna A RAM ==
  I : Inport
  Q : Inport  Port=2
  We : Inport  Port=3
  Wr Addr : Inport  Port=4
  Rd Addr : Inport  Port=5
  Constant1 : xbsIndex_r4/Constant  arith_type=Signed (2's comp)  const=0  n_bits=14  bin_pt=13  explicit_period=on  period=1  equ=P=C  opselect=C  inp2=PCIN>>17  opr=+  inp1=P  carry=CIN  dbl_ovrd=off  block_type=constant
      settings: block_version=VER_STRING_GOES_HERE
  Constant8 : xbsIndex_r4/Constant  arith_type=Boolean  const=0  n_bits=1  bin_pt=0  explicit_period=off  period=1  equ=P=C  opselect=C  inp2=PCIN>>17  opr=+  inp1=P  carry=CIN  dbl_ovrd=off  block_type=constant
      settings: block_version=VER_STRING_GOES_HERE
  Dual Port RAM : xbsIndex_r4/Dual Port RAM  depth=2*numSubcarriers  initVector=[0.01:0.01:0.80 ones(1,48)]  distributed_mem=Block RAM  init_a=0  init_b=0  rst_a=off  rst_b=off  en_a=off  en_b=off  latency=1  write_mode_A=Read After Write  write_mode_B=Read After Write  dbl_ovrd=off  optimize=Area  use_rpm=off  block_type=dpram
      settings: xl_area=[0 0 1 0 0 0 0]  block_version=VER_STRING_GOES_HERE
  Dual Port RAM1 : xbsIndex_r4/Dual Port RAM  depth=2*numSubcarriers  initVector=[0.80:-0.01:0.01 ones(1,48)]  distributed_mem=Block RAM  init_a=0  init_b=0  rst_a=off  rst_b=off  en_a=off  en_b=off  latency=1  write_mode_A=Read After Write  write_mode_B=Read After Write  dbl_ovrd=off  optimize=Area  use_rpm=off  block_type=dpram
      settings: xl_area=[0 0 1 0 0 0 0]  block_version=VER_STRING_GOES_HERE
  " I" : Outport
  " Q" : Outport  Port=2
  wires:
  Wr Addr/1 -> Dual Port RAM1/1, Dual Port RAM/1
  Constant8/1 -> Dual Port RAM/6, Dual Port RAM1/6
  Rd Addr/1 -> Dual Port RAM1/4, Dual Port RAM/4
  Dual Port RAM1/2 -> " Q"/1
  Dual Port RAM/2 -> " I"/1
  I/1 -> Dual Port RAM/2
  Q/1 -> Dual Port RAM1/2
  We/1 -> Dual Port RAM1/3, Dual Port RAM/3
  Constant1/1 -> Dual Port RAM/5, Dual Port RAM1/5

== system ofdm_txrx_mimo_2x/OFDM Rx MIMO/FFT & Chan Est/Input Buffers/Antenna B RAM ==
  I : Inport
  Q : Inport  Port=2
  We : Inport  Port=3
  Wr Addr : Inport  Port=4
  Rd Addr : Inport  Port=5
  Constant1 : xbsIndex_r4/Constant  arith_type=Signed (2's comp)  const=0  n_bits=14  bin_pt=13  explicit_period=on  period=1  equ=P=C  opselect=C  inp2=PCIN>>17  opr=+  inp1=P  carry=CIN  dbl_ovrd=off  block_type=constant
      settings: block_version=VER_STRING_GOES_HERE
  Constant8 : xbsIndex_r4/Constant  arith_type=Boolean  const=0  n_bits=1  bin_pt=0  explicit_period=off  period=1  equ=P=C  opselect=C  inp2=PCIN>>17  opr=+  inp1=P  carry=CIN  dbl_ovrd=off  block_type=constant
      settings: block_version=VER_STRING_GOES_HERE
  Dual Port RAM : xbsIndex_r4/Dual Port RAM  depth=2*numSubcarriers  initVector=[0.01:0.01:0.80 ones(1,48)]  distributed_mem=Block RAM  init_a=0  init_b=0  rst_a=off  rst_b=off  en_a=off  en_b=off  latency=1  write_mode_A=Read After Write  write_mode_B=Read After Write  dbl_ovrd=off  optimize=Area  use_rpm=off  block_type=dpram
      settings: xl_area=[0 0 1 0 0 0 0]  block_version=VER_STRING_GOES_HERE
  Dual Port RAM1 : xbsIndex_r4/Dual Port RAM  depth=2*numSubcarriers  initVector=[0.80:-0.01:0.01 ones(1,48)]  distributed_mem=Block RAM  init_a=0  init_b=0  rst_a=off  rst_b=off  en_a=off  en_b=off  latency=1  write_mode_A=Read After Write  write_mode_B=Read After Write  dbl_ovrd=off  optimize=Area  use_rpm=off  block_type=dpram
      settings: xl_area=[0 0 1 0 0 0 0]  block_version=VER_STRING_GOES_HERE
  " I" : Outport
  " Q" : Outport  Port=2
  wires:
  We/1 -> Dual Port RAM/3, Dual Port RAM1/3
  Q/1 -> Dual Port RAM1/2
  I/1 -> Dual Port RAM/2
  Dual Port RAM/2 -> " I"/1
  Dual Port RAM1/2 -> " Q"/1
  Rd Addr/1 -> Dual Port RAM/4, Dual Port RAM1/4
  Constant8/1 -> Dual Port RAM1/6, Dual Port RAM/6
  Wr Addr/1 -> Dual Port RAM/1, Dual Port RAM1/1
  Constant1/1 -> Dual Port RAM/5, Dual Port RAM1/5

== system ofdm_txrx_mimo_2x/OFDM Rx MIMO/FFT & Chan Est/Output Buffers/AntA Buffer ==
  I : Inport
  Q : Inport  Port=2
  We : Inport  Port=3
  Wr Addr : Inport  Port=4
  Rd Addr : Inport  Port=5
  Constant : xbsIndex_r4/Constant  arith_type=Signed (2's comp)  const=0  n_bits=16  bin_pt=15  explicit_period=on  period=2  equ=P=C  opselect=C  inp2=PCIN>>17  opr=+  inp1=P  carry=CIN  dbl_ovrd=off  block_type=constant
      settings: block_version=10.1.2
  Constant8 : xbsIndex_r4/Constant  arith_type=Boolean  const=0  n_bits=1  bin_pt=0  explicit_period=off  period=1  equ=P=C  opselect=C  inp2=PCIN>>17  opr=+  inp1=P  carry=CIN  dbl_ovrd=off  block_type=constant
      settings: block_version=VER_STRING_GOES_HERE
  Dual Port RAM : xbsIndex_r4/Dual Port RAM  depth=numSubcarriers  initVector=[0.01:0.01:0.80 ones(1,48)]  distributed_mem=Block RAM  init_a=0  init_b=0  rst_a=off  rst_b=off  en_a=off  en_b=off  latency=1  write_mode_A=Read After Write  write_mode_B=Read After Write  dbl_ovrd=off  optimize=Area  use_rpm=off  block_type=dpram
      settings: xl_area=[0 0 1 0 0 0 0]  block_version=VER_STRING_GOES_HERE
  Dual Port RAM1 : xbsIndex_r4/Dual Port RAM  depth=numSubcarriers  initVector=[0.80:-0.01:0.01 ones(1,48)]  distributed_mem=Block RAM  init_a=0  init_b=0  rst_a=off  rst_b=off  en_a=off  en_b=off  latency=1  write_mode_A=Read After Write  write_mode_B=Read After Write  dbl_ovrd=off  optimize=Area  use_rpm=off  block_type=dpram
      settings: xl_area=[0 0 1 0 0 0 0]  block_version=VER_STRING_GOES_HERE
  " I" : Outport
  " Q" : Outport  Port=2
  wires:
  We/1 -> Dual Port RAM/3, Dual Port RAM1/3
  Q/1 -> Dual Port RAM1/2
  I/1 -> Dual Port RAM/2
  Dual Port RAM/2 -> " I"/1
  Dual Port RAM1/2 -> " Q"/1
  Rd Addr/1 -> Dual Port RAM/4, Dual Port RAM1/4
  Constant8/1 -> Dual Port RAM1/6, Dual Port RAM/6
  Wr Addr/1 -> Dual Port RAM/1, Dual Port RAM1/1
  Constant/1 -> Dual Port RAM/5, Dual Port RAM1/5

== system ofdm_txrx_mimo_2x/OFDM Rx MIMO/FFT & Chan Est/Output Buffers/AntB Buffer ==
  I : Inport
  Q : Inport  Port=2
  We : Inport  Port=3
  Wr Addr : Inport  Port=4
  Rd Addr : Inport  Port=5
  Constant : xbsIndex_r4/Constant  arith_type=Signed (2's comp)  const=0  n_bits=16  bin_pt=15  explicit_period=on  period=2  equ=P=C  opselect=C  inp2=PCIN>>17  opr=+  inp1=P  carry=CIN  dbl_ovrd=off  block_type=constant
      settings: block_version=10.1.2
  Constant8 : xbsIndex_r4/Constant  arith_type=Boolean  const=0  n_bits=1  bin_pt=0  explicit_period=off  period=1  equ=P=C  opselect=C  inp2=PCIN>>17  opr=+  inp1=P  carry=CIN  dbl_ovrd=off  block_type=constant
      settings: block_version=VER_STRING_GOES_HERE
  Dual Port RAM : xbsIndex_r4/Dual Port RAM  depth=numSubcarriers  initVector=[0.01:0.01:0.80 ones(1,48)]  distributed_mem=Block RAM  init_a=0  init_b=0  rst_a=off  rst_b=off  en_a=off  en_b=off  latency=1  write_mode_A=Read After Write  write_mode_B=Read After Write  dbl_ovrd=off  optimize=Area  use_rpm=off  block_type=dpram
      settings: xl_area=[0 0 1 0 0 0 0]  block_version=VER_STRING_GOES_HERE
  Dual Port RAM1 : xbsIndex_r4/Dual Port RAM  depth=numSubcarriers  initVector=[0.80:-0.01:0.01 ones(1,48)]  distributed_mem=Block RAM  init_a=0  init_b=0  rst_a=off  rst_b=off  en_a=off  en_b=off  latency=1  write_mode_A=Read After Write  write_mode_B=Read After Write  dbl_ovrd=off  optimize=Area  use_rpm=off  block_type=dpram
      settings: xl_area=[0 0 1 0 0 0 0]  block_version=VER_STRING_GOES_HERE
  " I" : Outport
  " Q" : Outport  Port=2
  wires:
  Wr Addr/1 -> Dual Port RAM1/1, Dual Port RAM/1
  Constant8/1 -> Dual Port RAM/6, Dual Port RAM1/6
  Rd Addr/1 -> Dual Port RAM1/4, Dual Port RAM/4
  Dual Port RAM1/2 -> " Q"/1
  Dual Port RAM/2 -> " I"/1
  I/1 -> Dual Port RAM/2
  Q/1 -> Dual Port RAM1/2
  We/1 -> Dual Port RAM1/3, Dual Port RAM/3
  Constant/1 -> Dual Port RAM/5, Dual Port RAM1/5

== system ofdm_txrx_mimo_2x/OFDM Rx MIMO/FFT & Chan Est/Symbol Counter/S-R Latch ==
  S : Inport
  R : Inport  Port=2
  Constant2 : xbsIndex_r4/Constant  arith_type=Boolean  const=1  n_bits=1  bin_pt=0  explicit_period=off  period=1  equ=P=C  opselect=C  inp2=PCIN>>17  opr=+  inp1=P  carry=CIN  dbl_ovrd=off  block_type=constant
      settings: block_version=VER_STRING_GOES_HERE
  Register : xbsIndex_r4/Register  init=0  rst=on  en=on  dbl_ovrd=off  block_type=register
      settings: xl_area=[0, 0, 0, 0, 0, 0, 0]  block_version=VER_STRING_GOES_HERE
  D : Outport
  wires:
  Register/1 -> D/1
  S/1 -> Register/3
  R/1 -> Register/2
  Constant2/1 -> Register/1

== system ofdm_txrx_mimo_2x/OFDM Rx MIMO/Long Corr 4x/C1/C1 ==
  xn : Inport
  DAddr : Inport  Port=2
  CAddr : Inport  Port=3
  LD : Inport  Port=4
  Down Sample2 : xbsIndex_r4/Down Sample  sample_ratio=length(coef2)  sample_phase=First Value of Frame  en=off  latency=0  dbl_ovrd=off  block_type=dsamp
      settings: xl_area=[5 5 0 4 0 0 0]  block_version=VER_STRING_GOES_HERE
  MAC : Subsystem
  R : xbsIndex_r4/Addressable Shift Register  infer_latency=off  depth=length(coef2)  initVector=[0]  en=off  dbl_ovrd=off  use_behavioral_HDL=off  use_rpm=off  block_type=addrsr
      settings: infoedit="Delay of  configurable length.  Any element  in the delay     line   can    be   addressed   and   driven    onto   the   output     port.<P><P>Hardware  notes: Implemented  using SRL16s.     If Virtex-4, Virtex-II or Spartan-3 devices are used, multiple SRLC16s are cascaded together."  xl_area=[1 0 0 2 0 0 0]  block_version=VER_STRING_GOES_HERE
  ROM1 : xbsIndex_r4/ROM  depth=length(coef2)  initVector=coef2  distributed_mem=Distributed memory  rst=off  init_reg=0  en=off  latency=1  arith_type=Unsigned  n_bits=1  bin_pt=0  dbl_ovrd=off  optimize=Area  use_rpm=off  block_type=sprom
      settings: xl_area=[1 1 0 1 0 0 0]  block_version=VER_STRING_GOES_HERE
  yn : Outport
  xnz : Outport  Port=2
  wires:
  LD/1 -> MAC/3(ld)
  ROM1/1 -> MAC/2(coeff)
  Down Sample2/1 -> yn/1
  MAC/1(yn) -> Down Sample2/1
  R/1 -> MAC/1(a), xnz/1
  CAddr/1 -> ROM1/1
  DAddr/1 -> R/2
  xn/1 -> R/1

== system ofdm_txrx_mimo_2x/OFDM Rx MIMO/Long Corr 4x/C1/C10 ==
  xn : Inport
  DAddr : Inport  Port=2
  CAddr : Inport  Port=3
  LD : Inport  Port=4
  Down Sample1 : xbsIndex_r4/Down Sample  sample_ratio=length(coef2)  sample_phase=First Value of Frame  en=off  latency=0  dbl_ovrd=off  block_type=dsamp
      settings: xl_area=[3 3 0 2 0 0 0]  block_version=VER_STRING_GOES_HERE
  Down Sample2 : xbsIndex_r4/Down Sample  sample_ratio=length(coef2)  sample_phase=First Value of Frame  en=off  latency=0  dbl_ovrd=off  block_type=dsamp
      settings: xl_area=[5 5 0 4 0 0 0]  block_version=VER_STRING_GOES_HERE
  MAC : Subsystem
  R : xbsIndex_r4/Addressable Shift Register  infer_latency=off  depth=length(coef2)  initVector=[0]  en=off  dbl_ovrd=off  use_behavioral_HDL=off  use_rpm=off  block_type=addrsr
      settings: infoedit="Delay of  configurable length.  Any element  in the delay     line   can    be   addressed   and   driven    onto   the   output     port.<P><P>Hardware  notes: Implemented  using SRL16s.     If Virtex-4, Virtex-II or Spartan-3 devices are used, multiple SRLC16s are cascaded together."  xl_area=[1 0 0 2 0 0 0]  block_version=VER_STRING_GOES_HERE
  ROM1 : xbsIndex_r4/ROM  depth=length(coef2)  initVector=coef2  distributed_mem=Distributed memory  rst=off  init_reg=0  en=off  latency=1  arith_type=Unsigned  n_bits=1  bin_pt=0  dbl_ovrd=off  optimize=Area  use_rpm=off  block_type=sprom
      settings: xl_area=[1 1 0 1 0 0 0]  block_version=VER_STRING_GOES_HERE
  yn : Outport
  xnz : Outport  Port=2
  wires:
  LD/1 -> MAC/3(ld)
  ROM1/1 -> MAC/2(coeff)
  Down Sample2/1 -> yn/1
  MAC/1(yn) -> Down Sample2/1
  R/1 -> MAC/1(a), xnz/1
  CAddr/1 -> ROM1/1
  DAddr/1 -> R/2
  xn/1 -> Down Sample1/1
  Down Sample1/1 -> R/1
